simulink model "HvacEvaprTCtrl"  (R2022b)
settings: Solver=FixedStepDiscrete  StartTime=0.0  StopTime=10.0  FixedStep=1  RelTol=1e-3
settings Model: Creator=blue.ge  LastModifiedBy=blue.ge
settings DataIOCC: SaveFormat=StructureWithTime
settings OptimizationCC: DefaultParameterBehavior=Inlined  EnhancedBackFolding=on  PassReuseOutputArgsAs=Individual arguments  ZeroExternalMemoryAtStartup=off  ZeroInternalMemoryAtStartup=off  OptimizeBlockOrder=speed  DifferentSizesBufferReuse=on  OptimizationCustomize=off  EfficientTunableParamExpr=on
settings DebuggingCC: ParameterTunabilityLossMsg=error
settings ModelReferenceCC: ModelReferenceNumInstancesAllowed=Single
settings SFSimCC: MATLABDynamicMemAlloc=off
settings RTWCC: Description=AUTOSAR  SystemTargetFile=autosar.tlc  GenCodeOnly=on  TemplateMakefile=ert_default_tmf
settings CodeAppCC: ForceParamTrailComments=on  IgnoreCustomStorageClasses=off  OperatorAnnotations=on  SimulinkDataObjDesc=on  SFDataObjDesc=on  InsertBlockDesc=on
settings STFCustomTargetCC: TargetLangStandard=C99 (ISO)  MultiwordLength=256  ParMdlRefBuildCompliant=on  CompOptLevelCompliant=on  IncludeMdlTerminateFcn=off  CombineOutputUpdateFcns=on  SuppressErrorStatus=on  ERTCustomFileBanners=on  SupportNonFinite=off  SupportComplex=off  SupportContinuousTime=off  SupportNonInlinedSFcns=off  RemoveResetFunc=on  MATLABClassNameForMDSCustomization=Simulink.SoftwareTarget.ERTCustomization  AutosarCompliant=on  UseToolchainInfoCompliant=on  LookupTableObjectStructAxisOrder=2,1,3,4,...  LUTObjectStructOrderEvenSpacing=Size,Table,Breakpoints  SystemTargetFile=autosar.tlc
settings ERTTargetCC: TargetLangStandard=C99 (ISO)  ConcurrentExecutionCompliant=off  IncludeMdlTerminateFcn=off  SuppressErrorStatus=on  SupportNonFinite=off  SupportComplex=off  ModelStepFunctionPrototypeControlCompliant=off  CPPClassGenCompliant=off  AutosarCompliant=on  LookupTableObjectStructAxisOrder=2,1,3,4,...  LUTObjectStructOrderEvenSpacing=Size,Table,Breakpoints  InlinedParameterPlacement=NonHierarchical  ERTCustomFileTemplate=example_file_process.tlc

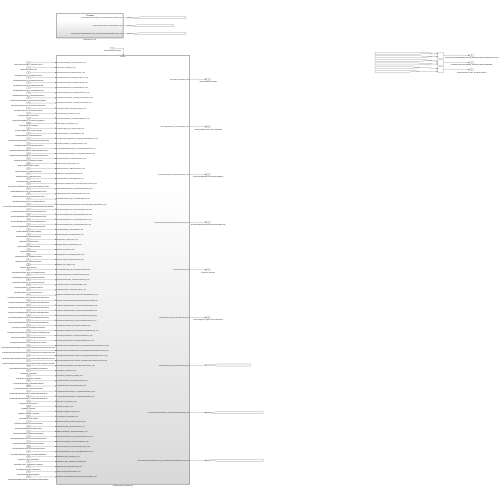
[thumbnail: Simulink's stored preview of the top-level diagram]
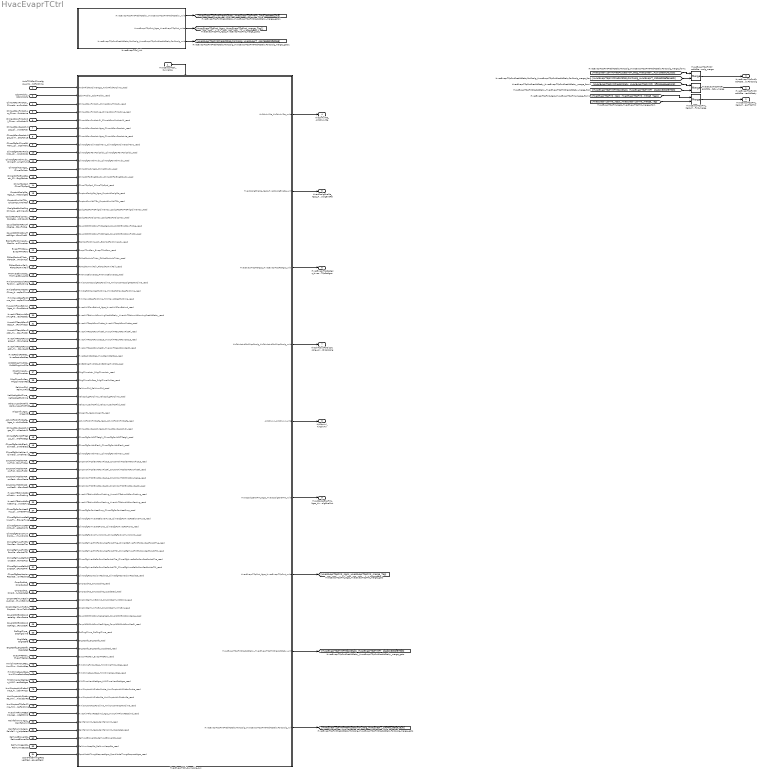
== system HvacEvaprTCtrl ==
  HvacEvaprTCtrl_Runnable : Inport  SampleTime=1  OutputFunctionCall=on
  AmbTFildForClimaNgcc_AmbTFildForClima : Inport  Port=2  OutDataTypeStr=T30  PortDimensions=1
  CdsnProblty_CdsnProblty : Inport  Port=3  OutDataTypeStr=Fac30  PortDimensions=1
  ClimaAtRowFrntActv_ClimaAtRowFrntActv : Inport  Port=4  OutDataTypeStr=Boolean  PortDimensions=1
  ClimaAtRowFrntActvLe_ClimaAtRowFrntActvLe : Inport  Port=5  OutDataTypeStr=Boolean  PortDimensions=1
  ClimaAtRowFrntActvRi_ClimaAtRowFrntActvRi : Inport  Port=6  OutDataTypeStr=Boolean  PortDimensions=1
  ClimaAtRowSecActvNgcc_ClimaAtRowSecActv : Inport  Port=7  OutDataTypeStr=Boolean  PortDimensions=1
  ClimaAtRowSecActvNgcc_ClimaAtRowSecActvLe : Inport  Port=8  OutDataTypeStr=Boolean  PortDimensions=1
  ClimaCfgForClimaCtrlMan1_ClimaCfgForClimaCtrlMan1 : Inport  Port=9  OutDataTypeStr=Boolean  PortDimensions=1
  ClimaCfgForFanPotiCtrld1_ClimaCfgForFanPotiCtrld1 : Inport  Port=10  OutDataTypeStr=Boolean  PortDimensions=1
  ClimaCfgForVehHyb1_ClimaCfgForVehHyb1 : Inport  Port=11  OutDataTypeStr=Boolean  PortDimensions=1
  ClimaCtrlActvNgcc_ClimaCtrlActv : Inport  Port=12  OutDataTypeStr=Boolean  PortDimensions=1
  ClimaCtrlForEngStbActv_ClimaCtrlForEngStbActv : Inport  Port=13  OutDataTypeStr=Boolean  PortDimensions=1
  ClimaTSpSpcl_ClimaTSpSpcl : Inport  Port=14  OutDataTypeStr=Bus: ClimaTSpSpcl  PortDimensions=1  BusOutputAsStruct=on
  CmptmtCoolgSts_Ngcc_CmptmtCoolgSts : Inport  Port=15  OutDataTypeStr=Enum: CmptmtCoolgSts  PortDimensions=1
  CmptmtSunMtrlTErr_CmptmtSunMtrlTErr : Inport  Port=16  OutDataTypeStr=T30  PortDimensions=1
  CoolgFastForPrePrkgClimaActv_CoolgFastForPrePrkgClimaActv : Inport  Port=17  OutDataTypeStr=Boolean  PortDimensions=1
  CoolgReqForDispActv_CoolgReqForDispActv : Inport  Port=18  OutDataTypeStr=Enum: CoolgReqForDisp  PortDimensions=1
  CoupldCtrlErrAtRowFirstLeNgcc_CoupldCtrlErrAtRowFirstLe : Inport  Port=19  OutDataTypeStr=T30  PortDimensions=1
  CoupldCtrlErrAtRowFirstRiNgcc_CoupldCtrlErrAtRowFirstRi : Inport  Port=20  OutDataTypeStr=T30  PortDimensions=1
  EcoModForClimaActv_EcoModForClimaActv : Inport  Port=21  OutDataTypeStr=Enum: OnOff1  PortDimensions=1
  EvaprTFrntRaw_EvaprTFrntRaw : Inport  Port=22  OutDataTypeStr=Bus: EvaprTFrnt  PortDimensions=1  BusOutputAsStruct=on
  FltModForAmbTActv_FltModForAmbTActv : Inport  Port=23  OutDataTypeStr=Boolean  PortDimensions=1
  FltModForHmiFailr_FltModForHmiFailr : Inport  Port=24  OutDataTypeStr=Boolean  PortDimensions=1
  FrntHvacBlowerSts_FrntHvacBlowerSts : Inport  Port=25  OutDataTypeStr=Enum: HvacFanSts  PortDimensions=1
  HmiCmptmtCoolgReqForClima_HmiCmptmtCoolgReqForClima : Inport  Port=26  OutDataTypeStr=Enum: HmiCmptmtCoolgReq  PortDimensions=1
  HmiDefrstMaxReqForClima_HmiDefrstMaxReqForClima : Inport  Port=27  OutDataTypeStr=Enum: ActrReq  PortDimensions=1
  HmiMaxACReqForClima_HmiMaxACReqForClima : Inport  Port=28  OutDataTypeStr=Enum: OnOff1  PortDimensions=1
  HvacAirMFlowEstimd_Ngcc_HvacAirMFlowEstimd : Inport  Port=29  OutMin=0  OutMax=1000  OutDataTypeStr=MFlow1  PortDimensions=1
  HvacAirTEstimnAtRowAvgPredAtStaby_HvacAirTEstimnAtRowAvgPredAtStaby : Inport  Port=30  OutDataTypeStr=T30  PortDimensions=1
  HvacAirTReqAtRowFirstLe_HvacAirTReqAtRowFirstLe : Inport  Port=31  OutDataTypeStr=T30  PortDimensions=1
  HvacAirTReqAtRowFirstRi_HvacAirTReqAtRowFirstRi : Inport  Port=32  OutDataTypeStr=T30  PortDimensions=1
  HvacAirTReqAtRowSecLe_HvacAirTReqAtRowSecLe : Inport  Port=33  OutDataTypeStr=T30  PortDimensions=1
  HvacAirTReqAtRowSecRi_HvacAirTReqAtRowSecRi : Inport  Port=34  OutDataTypeStr=T30  PortDimensions=1
  HvacRecircRatReq_HvacRecircRatReq : Inport  Port=35  OutDataTypeStr=Perc30  PortDimensions=1
  OnBdChrgrHndlSts_OnBdChrgrHndlSts : Inport  Port=36  OutDataTypeStr=Enum: OnBdChrgrSts1  PortDimensions=1
  PrkgClimaActv_PrkgClimaActv : Inport  Port=37  OutDataTypeStr=Enum: PrkgClimaActv  PortDimensions=1
  PrkgClimaOnReq_PrkgClimaOnReq : Inport  Port=38  OutDataTypeStr=Boolean  PortDimensions=1
  RelHumFild_RelHumFild : Inport  Port=39  OutDataTypeStr=Perc7  PortDimensions=1
  VehSpdLgtForClima_VehSpdLgtForClima : Inport  Port=40  OutDataTypeStr=Bus: VehSpdLgtForClima  PortDimensions=1  BusOutputAsStruct=on
  VehSunLoadTotFild_VehSunLoadTotFild : Inport  Port=41  OutDataTypeStr=SunLoad30  PortDimensions=1
  WipgInfo_Ngcc_WipgInfo : Inport  Port=42  OutDataTypeStr=Bus: WipgInfo  PortDimensions=1  BusOutputAsStruct=on
  ActvnOfWshrFrntSafe_Ngcc_ActvnOfWshrFrntSafe : Inport  Port=43  OutDataTypeStr=Enum: OnOffCrit1  PortDimensions=1
  ClimaAtRowSecActvNgcc_ClimaAtRowSecActvRi : Inport  Port=44  OutDataTypeStr=Boolean  PortDimensions=1
  ClimaCfgForNrOfTSetg1_ClimaCfgForNrOfTSetg1 : Inport  Port=45  OutDataTypeStr=Enum: ClimaCfgForNrOfTSetg1  PortDimensions=1
  ClimaCfgForVehElec1_ClimaCfgForVehElec1 : Inport  Port=46  OutDataTypeStr=Boolean  PortDimensions=1
  ClimaCfgForVehHev1_ClimaCfgForVehHev1 : Inport  Port=47  OutDataTypeStr=Boolean  PortDimensions=1
  CmptmtAirTCtrlErrAtRowFirstLe_CmptmtAirTCtrlErrAtRowFirstLe : Inport  Port=48  OutDataTypeStr=T30  PortDimensions=1
  CmptmtAirTCtrlErrAtRowFirstRi_CmptmtAirTCtrlErrAtRowFirstRi : Inport  Port=49  OutDataTypeStr=T30  PortDimensions=1
  CmptmtAirTCtrlErrAtRowSecLe_CmptmtAirTCtrlErrAtRowSecLe : Inport  Port=50  OutDataTypeStr=T30  PortDimensions=1
  CmptmtAirTCtrlErrAtRowSecRi_CmptmtAirTCtrlErrAtRowSecRi : Inport  Port=51  OutDataTypeStr=T30  PortDimensions=1
  HvacAirTEstimdAtRowFirstAvg_HvacAirTEstimdAtRowFirstAvg : Inport  Port=52  OutDataTypeStr=T30  PortDimensions=1
  HvacAirTEstimdAtRowSecAvg_HvacAirTEstimdAtRowSecAvg : Inport  Port=53  OutDataTypeStr=T30  PortDimensions=1
  ClimaCfgForForHeatPmp_ClimaCfgForForHeatPmp : Inport  Port=54  OutDataTypeStr=Enum: CarCfgForHeatPmpTyp  PortDimensions=1
  ClimaCfgForHvacReBlowerType_ClimaCfgForHvacReBlowerType : Inport  Port=55  OutDataTypeStr=Enum: ClimaCfgForHvacReBlowerType  PortDimensions=1
  ClimaCfgForHvacRePrsnt1_ClimaCfgForHvacRePrsnt1 : Inport  Port=56  OutDataTypeStr=Enum: CarCfgForHvacRe  PortDimensions=1
  ClimaCfgForSnsrHumPrsnt1_ClimaCfgForSnsrHumPrsnt1 : Inport  Port=57  OutDataTypeStr=Boolean  PortDimensions=1
  ClimaCfgHvacFrntForRowSecForActrTLe_ClimaCfgHvacFrntForRowSecForActrTLe : Inport  Port=58  OutDataTypeStr=Boolean  PortDimensions=1
  ClimaCfgHvacFrntForRowSecForActrTRi_ClimaCfgHvacFrntForRowSecForActrTRi : Inport  Port=59  OutDataTypeStr=Boolean  PortDimensions=1
  ClimaCfgHvacReForRowSecForActrTLe_ClimaCfgHvacReForRowSecForActrTLe : Inport  Port=60  OutDataTypeStr=UInt8  PortDimensions=1
  ClimaCfgHvacReForRowSecForActrTRi_ClimaCfgHvacReForRowSecForActrTRi : Inport  Port=61  OutDataTypeStr=UInt8  PortDimensions=1
  ClimaCfgReqVersionReplace_ClimaCfgReqVersionReplace : Inport  Port=62  OutDataTypeStr=UInt8  PortDimensions=1
  CmprSpdAct_CmprSpdAct : Inport  Port=63  OutDataTypeStr=CmprSpd1  PortDimensions=1
  CmprSpdAct_CmprSpdAct_IsUpdated : Inport  Port=64  OutDataTypeStr=boolean  PortDimensions=1
  CmptmtRelHumEstimd_CmptmtRelHumEstimd : Inport  Port=65  OutDataTypeStr=Perc7  PortDimensions=1
  CmptmtRelHumToExt_CmptmtRelHumToExt : Inport  Port=66  OutDataTypeStr=Bus: CmptmtRelHumToExt  PortDimensions=1  BusOutputAsStruct=on
  CoupldCtrlErrAtRowSecLeNgcc_CoupldCtrlErrAtRowSecLe : Inport  Port=67  OutDataTypeStr=T30  PortDimensions=1
  CoupldCtrlErrAtRowSecRiNgcc_CoupldCtrlErrAtRowSecRi : Inport  Port=68  OutDataTypeStr=T30  PortDimensions=1
  DsChrgClima_DsChrgClima : Inport  Port=69  OutDataTypeStr=Boolean  PortDimensions=1
  EngNSafe_EngNSafe : Inport  Port=70  OutDataTypeStr=Bus: EngNSafe  PortDimensions=1  BusOutputAsStruct=on
  EngNSafe_EngNSafe_IsUpdated : Inport  Port=71  OutDataTypeStr=boolean  PortDimensions=1
  EvaprTReRaw_EvaprTReRaw : Inport  Port=72  OutDataTypeStr=Bus: EvaprTFrnt  PortDimensions=1  BusOutputAsStruct=on
  HmiClimaFrntAutReq_HmiClimaFrntAutReq : Inport  Port=73  OutDataTypeStr=Enum: OnOff1  PortDimensions=1
  HmiClimaReAutReq_HmiClimaReAutReq : Inport  Port=74  OutDataTypeStr=Enum: OnOff1  PortDimensions=1
  HMIClimaVentSetNgcc_HMIClimaVentSetNgcc : Inport  Port=75  OutDataTypeStr=Enum: OnOff1  PortDimensions=1
  HmiCmptmtAirDistbnFrntLe_HmiCmptmtAirDistbnFrntLe : Inport  Port=76  OutDataTypeStr=Enum: HmiCmptmtAirDistbnFrnt  PortDimensions=1
  HmiCmptmtAirDistbnRe_HmiCmptmtAirDistbnRe : Inport  Port=77  OutDataTypeStr=Enum: HmiCmptmtAirDistbnFrnt  PortDimensions=1
  HmiCmptmtTSpForClima_HmiCmptmtTSpForClima : Inport  Port=78  OutDataTypeStr=Bus: HmiCmptmtTSp  PortDimensions=1  BusOutputAsStruct=on
  HvacAirMFlowReEstimd_Ngcc_HvacAirMFlowReEstimd : Inport  Port=79  OutMin=0  OutMax=1000  OutDataTypeStr=MFlow1  PortDimensions=1
  RainfallAmnt_Ngcc_RainfallAmnt : Inport  Port=80  OutDataTypeStr=Enum: AmntSnsr  PortDimensions=1
  RainfallAmnt_Ngcc_RainfallAmnt_IsUpdated : Inport  Port=81  OutDataTypeStr=boolean  PortDimensions=1
  ReHvacBlowerSts_ReHvacBlowerSts : Inport  Port=82  OutDataTypeStr=Enum: HvacFanSts  PortDimensions=1
  RelHumKeepSts_RelHumKeepSts : Inport  Port=83  OutDataTypeStr=Enum: RelHumKeepSts  PortDimensions=1
  SportModeThmgtRequestNgcc_SportModeThmgtRequestNgcc : Inport  Port=84  OutDataTypeStr=Enum: ThmgtLevel  PortDimensions=1
  HvacEvaprTCtrl_Init : Subsystem
  HvacEvaprTCtrl_Runnable_sys : Subsystem
  HvacEvaprTSpFrntPredAtStabyForCoolg_HvacEvaprTSpFrntPredAtStabyForCoolg_merge : Merge
  HvacEvaprTSpFrntPredAtStaby_HvacEvaprTSpFrntPredAtStaby_merge : Merge
  HvacEvaprTSpFrnt_Ngcc_HvacEvaprTSpFrnt_merge : Merge
  HvacEvaprTSpFrntPredAtStaby_HvacEvaprTSpFrntPredAtStaby : Outport  OutDataTypeStr=HvacEvaprTSp  PortDimensions=1
  HvacEvaprTSpFrnt_Ngcc_HvacEvaprTSpFrnt : Outport  Port=2  OutDataTypeStr=HvacEvaprTSp  PortDimensions=1
  HvacEvaprTSpFrntPredAtStabyForCoolg_HvacEvaprTSpFrntPredAtStabyForCoolg : Outport  Port=3  OutDataTypeStr=HvacEvaprTSp  PortDimensions=1
  AirCdnAvlRe_AirCdnAvlRe : Outport  Port=4  OutDataTypeStr=Boolean  PortDimensions=1
  HvacCoolgEnaRe_Ngcc_HvacCoolgEnaRe : Outport  Port=5  OutDataTypeStr=Enum: OnOff1  PortDimensions=1
  HvacEvaprTSpReNgcc_HvacEvaprTSpReNgcc : Outport  Port=6  OutDataTypeStr=HvacEvaprTSp  PortDimensions=1
  AirCdnActvdForDispCoolg_AirCdnActvdForDispCoolg : Outport  Port=7  OutDataTypeStr=Boolean  PortDimensions=1
  AirCdnAvl_AirCdnAvl : Outport  Port=8  OutDataTypeStr=Boolean  PortDimensions=1
  HvacCoolgEnaFrnt_Ngcc_HvacCoolgEnaFrnt : Outport  Port=9  OutDataTypeStr=Enum: OnOff1  PortDimensions=1
  wires:
  AmbTFildForClimaNgcc_AmbTFildForClima/1 -> HvacEvaprTCtrl_Runnable_sys/1(AmbTFildForClimaNgcc_AmbTFildForClima_read)
  CdsnProblty_CdsnProblty/1 -> HvacEvaprTCtrl_Runnable_sys/2(CdsnProblty_CdsnProblty_read)
  ClimaAtRowFrntActv_ClimaAtRowFrntActv/1 -> HvacEvaprTCtrl_Runnable_sys/3(ClimaAtRowFrntActv_ClimaAtRowFrntActv_read)
  ClimaAtRowFrntActvLe_ClimaAtRowFrntActvLe/1 -> HvacEvaprTCtrl_Runnable_sys/4(ClimaAtRowFrntActvLe_ClimaAtRowFrntActvLe_read)
  ClimaAtRowFrntActvRi_ClimaAtRowFrntActvRi/1 -> HvacEvaprTCtrl_Runnable_sys/5(ClimaAtRowFrntActvRi_ClimaAtRowFrntActvRi_read)
  ClimaAtRowSecActvNgcc_ClimaAtRowSecActv/1 -> HvacEvaprTCtrl_Runnable_sys/6(ClimaAtRowSecActvNgcc_ClimaAtRowSecActv_read)
  ClimaAtRowSecActvNgcc_ClimaAtRowSecActvLe/1 -> HvacEvaprTCtrl_Runnable_sys/7(ClimaAtRowSecActvNgcc_ClimaAtRowSecActvLe_read)
  ClimaCfgForClimaCtrlMan1_ClimaCfgForClimaCtrlMan1/1 -> HvacEvaprTCtrl_Runnable_sys/8(ClimaCfgForClimaCtrlMan1_ClimaCfgForClimaCtrlMan1_read)
  ClimaCfgForFanPotiCtrld1_ClimaCfgForFanPotiCtrld1/1 -> HvacEvaprTCtrl_Runnable_sys/9(ClimaCfgForFanPotiCtrld1_ClimaCfgForFanPotiCtrld1_read)
  ClimaCfgForVehHyb1_ClimaCfgForVehHyb1/1 -> HvacEvaprTCtrl_Runnable_sys/10(ClimaCfgForVehHyb1_ClimaCfgForVehHyb1_read)
  ClimaCtrlActvNgcc_ClimaCtrlActv/1 -> HvacEvaprTCtrl_Runnable_sys/11(ClimaCtrlActvNgcc_ClimaCtrlActv_read)
  ClimaCtrlForEngStbActv_ClimaCtrlForEngStbActv/1 -> HvacEvaprTCtrl_Runnable_sys/12(ClimaCtrlForEngStbActv_ClimaCtrlForEngStbActv_read)
  ClimaTSpSpcl_ClimaTSpSpcl/1 -> HvacEvaprTCtrl_Runnable_sys/13(ClimaTSpSpcl_ClimaTSpSpcl_read)
  CmptmtCoolgSts_Ngcc_CmptmtCoolgSts/1 -> HvacEvaprTCtrl_Runnable_sys/14(CmptmtCoolgSts_Ngcc_CmptmtCoolgSts_read)
  CmptmtSunMtrlTErr_CmptmtSunMtrlTErr/1 -> HvacEvaprTCtrl_Runnable_sys/15(CmptmtSunMtrlTErr_CmptmtSunMtrlTErr_read)
  CoolgFastForPrePrkgClimaActv_CoolgFastForPrePrkgClimaActv/1 -> HvacEvaprTCtrl_Runnable_sys/16(CoolgFastForPrePrkgClimaActv_CoolgFastForPrePrkgClimaActv_read)
  CoolgReqForDispActv_CoolgReqForDispActv/1 -> HvacEvaprTCtrl_Runnable_sys/17(CoolgReqForDispActv_CoolgReqForDispActv_read)
  CoupldCtrlErrAtRowFirstLeNgcc_CoupldCtrlErrAtRowFirstLe/1 -> HvacEvaprTCtrl_Runnable_sys/18(CoupldCtrlErrAtRowFirstLeNgcc_CoupldCtrlErrAtRowFirstLe_read)
  CoupldCtrlErrAtRowFirstRiNgcc_CoupldCtrlErrAtRowFirstRi/1 -> HvacEvaprTCtrl_Runnable_sys/19(CoupldCtrlErrAtRowFirstRiNgcc_CoupldCtrlErrAtRowFirstRi_read)
  EcoModForClimaActv_EcoModForClimaActv/1 -> HvacEvaprTCtrl_Runnable_sys/20(EcoModForClimaActv_EcoModForClimaActv_read)
  EvaprTFrntRaw_EvaprTFrntRaw/1 -> HvacEvaprTCtrl_Runnable_sys/21(EvaprTFrntRaw_EvaprTFrntRaw_read)
  FltModForAmbTActv_FltModForAmbTActv/1 -> HvacEvaprTCtrl_Runnable_sys/22(FltModForAmbTActv_FltModForAmbTActv_read)
  FltModForHmiFailr_FltModForHmiFailr/1 -> HvacEvaprTCtrl_Runnable_sys/23(FltModForHmiFailr_FltModForHmiFailr_read)
  FrntHvacBlowerSts_FrntHvacBlowerSts/1 -> HvacEvaprTCtrl_Runnable_sys/24(FrntHvacBlowerSts_FrntHvacBlowerSts_read)
  HmiCmptmtCoolgReqForClima_HmiCmptmtCoolgReqForClima/1 -> HvacEvaprTCtrl_Runnable_sys/25(HmiCmptmtCoolgReqForClima_HmiCmptmtCoolgReqForClima_read)
  HmiDefrstMaxReqForClima_HmiDefrstMaxReqForClima/1 -> HvacEvaprTCtrl_Runnable_sys/26(HmiDefrstMaxReqForClima_HmiDefrstMaxReqForClima_read)
  HmiMaxACReqForClima_HmiMaxACReqForClima/1 -> HvacEvaprTCtrl_Runnable_sys/27(HmiMaxACReqForClima_HmiMaxACReqForClima_read)
  HvacAirMFlowEstimd_Ngcc_HvacAirMFlowEstimd/1 -> HvacEvaprTCtrl_Runnable_sys/28(HvacAirMFlowEstimd_Ngcc_HvacAirMFlowEstimd_read)
  HvacAirTEstimnAtRowAvgPredAtStaby_HvacAirTEstimnAtRowAvgPredAtStaby/1 -> HvacEvaprTCtrl_Runnable_sys/29(HvacAirTEstimnAtRowAvgPredAtStaby_HvacAirTEstimnAtRowAvgPredAtStaby_read)
  HvacAirTReqAtRowFirstLe_HvacAirTReqAtRowFirstLe/1 -> HvacEvaprTCtrl_Runnable_sys/30(HvacAirTReqAtRowFirstLe_HvacAirTReqAtRowFirstLe_read)
  HvacAirTReqAtRowFirstRi_HvacAirTReqAtRowFirstRi/1 -> HvacEvaprTCtrl_Runnable_sys/31(HvacAirTReqAtRowFirstRi_HvacAirTReqAtRowFirstRi_read)
  HvacAirTReqAtRowSecLe_HvacAirTReqAtRowSecLe/1 -> HvacEvaprTCtrl_Runnable_sys/32(HvacAirTReqAtRowSecLe_HvacAirTReqAtRowSecLe_read)
  HvacAirTReqAtRowSecRi_HvacAirTReqAtRowSecRi/1 -> HvacEvaprTCtrl_Runnable_sys/33(HvacAirTReqAtRowSecRi_HvacAirTReqAtRowSecRi_read)
  HvacRecircRatReq_HvacRecircRatReq/1 -> HvacEvaprTCtrl_Runnable_sys/34(HvacRecircRatReq_HvacRecircRatReq_read)
  OnBdChrgrHndlSts_OnBdChrgrHndlSts/1 -> HvacEvaprTCtrl_Runnable_sys/35(OnBdChrgrHndlSts_OnBdChrgrHndlSts_read)
  PrkgClimaActv_PrkgClimaActv/1 -> HvacEvaprTCtrl_Runnable_sys/36(PrkgClimaActv_PrkgClimaActv_read)
  PrkgClimaOnReq_PrkgClimaOnReq/1 -> HvacEvaprTCtrl_Runnable_sys/37(PrkgClimaOnReq_PrkgClimaOnReq_read)
  RelHumFild_RelHumFild/1 -> HvacEvaprTCtrl_Runnable_sys/38(RelHumFild_RelHumFild_read)
  VehSpdLgtForClima_VehSpdLgtForClima/1 -> HvacEvaprTCtrl_Runnable_sys/39(VehSpdLgtForClima_VehSpdLgtForClima_read)
  VehSunLoadTotFild_VehSunLoadTotFild/1 -> HvacEvaprTCtrl_Runnable_sys/40(VehSunLoadTotFild_VehSunLoadTotFild_read)
  WipgInfo_Ngcc_WipgInfo/1 -> HvacEvaprTCtrl_Runnable_sys/41(WipgInfo_Ngcc_WipgInfo_read)
  ActvnOfWshrFrntSafe_Ngcc_ActvnOfWshrFrntSafe/1 -> HvacEvaprTCtrl_Runnable_sys/42(ActvnOfWshrFrntSafe_Ngcc_ActvnOfWshrFrntSafe_read)
  ClimaAtRowSecActvNgcc_ClimaAtRowSecActvRi/1 -> HvacEvaprTCtrl_Runnable_sys/43(ClimaAtRowSecActvNgcc_ClimaAtRowSecActvRi_read)
  ClimaCfgForNrOfTSetg1_ClimaCfgForNrOfTSetg1/1 -> HvacEvaprTCtrl_Runnable_sys/44(ClimaCfgForNrOfTSetg1_ClimaCfgForNrOfTSetg1_read)
  ClimaCfgForVehElec1_ClimaCfgForVehElec1/1 -> HvacEvaprTCtrl_Runnable_sys/45(ClimaCfgForVehElec1_ClimaCfgForVehElec1_read)
  ClimaCfgForVehHev1_ClimaCfgForVehHev1/1 -> HvacEvaprTCtrl_Runnable_sys/46(ClimaCfgForVehHev1_ClimaCfgForVehHev1_read)
  CmptmtAirTCtrlErrAtRowFirstLe_CmptmtAirTCtrlErrAtRowFirstLe/1 -> HvacEvaprTCtrl_Runnable_sys/47(CmptmtAirTCtrlErrAtRowFirstLe_CmptmtAirTCtrlErrAtRowFirstLe_read)
  CmptmtAirTCtrlErrAtRowFirstRi_CmptmtAirTCtrlErrAtRowFirstRi/1 -> HvacEvaprTCtrl_Runnable_sys/48(CmptmtAirTCtrlErrAtRowFirstRi_CmptmtAirTCtrlErrAtRowFirstRi_read)
  CmptmtAirTCtrlErrAtRowSecLe_CmptmtAirTCtrlErrAtRowSecLe/1 -> HvacEvaprTCtrl_Runnable_sys/49(CmptmtAirTCtrlErrAtRowSecLe_CmptmtAirTCtrlErrAtRowSecLe_read)
  CmptmtAirTCtrlErrAtRowSecRi_CmptmtAirTCtrlErrAtRowSecRi/1 -> HvacEvaprTCtrl_Runnable_sys/50(CmptmtAirTCtrlErrAtRowSecRi_CmptmtAirTCtrlErrAtRowSecRi_read)
  HvacAirTEstimdAtRowFirstAvg_HvacAirTEstimdAtRowFirstAvg/1 -> HvacEvaprTCtrl_Runnable_sys/51(HvacAirTEstimdAtRowFirstAvg_HvacAirTEstimdAtRowFirstAvg_read)
  HvacAirTEstimdAtRowSecAvg_HvacAirTEstimdAtRowSecAvg/1 -> HvacEvaprTCtrl_Runnable_sys/52(HvacAirTEstimdAtRowSecAvg_HvacAirTEstimdAtRowSecAvg_read)
  ClimaCfgForForHeatPmp_ClimaCfgForForHeatPmp/1 -> HvacEvaprTCtrl_Runnable_sys/53(ClimaCfgForForHeatPmp_ClimaCfgForForHeatPmp_read)
  ClimaCfgForHvacReBlowerType_ClimaCfgForHvacReBlowerType/1 -> HvacEvaprTCtrl_Runnable_sys/54(ClimaCfgForHvacReBlowerType_ClimaCfgForHvacReBlowerType_read)
  ClimaCfgForHvacRePrsnt1_ClimaCfgForHvacRePrsnt1/1 -> HvacEvaprTCtrl_Runnable_sys/55(ClimaCfgForHvacRePrsnt1_ClimaCfgForHvacRePrsnt1_read)
  ClimaCfgForSnsrHumPrsnt1_ClimaCfgForSnsrHumPrsnt1/1 -> HvacEvaprTCtrl_Runnable_sys/56(ClimaCfgForSnsrHumPrsnt1_ClimaCfgForSnsrHumPrsnt1_read)
  ClimaCfgHvacFrntForRowSecForActrTLe_ClimaCfgHvacFrntForRowSecForActrTLe/1 -> HvacEvaprTCtrl_Runnable_sys/57(ClimaCfgHvacFrntForRowSecForActrTLe_ClimaCfgHvacFrntForRowSecForActrTLe_read)
  ClimaCfgHvacFrntForRowSecForActrTRi_ClimaCfgHvacFrntForRowSecForActrTRi/1 -> HvacEvaprTCtrl_Runnable_sys/58(ClimaCfgHvacFrntForRowSecForActrTRi_ClimaCfgHvacFrntForRowSecForActrTRi_read)
  ClimaCfgHvacReForRowSecForActrTLe_ClimaCfgHvacReForRowSecForActrTLe/1 -> HvacEvaprTCtrl_Runnable_sys/59(ClimaCfgHvacReForRowSecForActrTLe_ClimaCfgHvacReForRowSecForActrTLe_read)
  ClimaCfgHvacReForRowSecForActrTRi_ClimaCfgHvacReForRowSecForActrTRi/1 -> HvacEvaprTCtrl_Runnable_sys/60(ClimaCfgHvacReForRowSecForActrTRi_ClimaCfgHvacReForRowSecForActrTRi_read)
  ClimaCfgReqVersionReplace_ClimaCfgReqVersionReplace/1 -> HvacEvaprTCtrl_Runnable_sys/61(ClimaCfgReqVersionReplace_ClimaCfgReqVersionReplace_read)
  CmprSpdAct_CmprSpdAct/1 -> HvacEvaprTCtrl_Runnable_sys/62(CmprSpdAct_CmprSpdAct_read)
  CmprSpdAct_CmprSpdAct_IsUpdated/1 -> HvacEvaprTCtrl_Runnable_sys/63(CmprSpdAct_CmprSpdAct_IsUpdated_read)
  CmptmtRelHumEstimd_CmptmtRelHumEstimd/1 -> HvacEvaprTCtrl_Runnable_sys/64(CmptmtRelHumEstimd_CmptmtRelHumEstimd_read)
  CmptmtRelHumToExt_CmptmtRelHumToExt/1 -> HvacEvaprTCtrl_Runnable_sys/65(CmptmtRelHumToExt_CmptmtRelHumToExt_read)
  CoupldCtrlErrAtRowSecLeNgcc_CoupldCtrlErrAtRowSecLe/1 -> HvacEvaprTCtrl_Runnable_sys/66(CoupldCtrlErrAtRowSecLeNgcc_CoupldCtrlErrAtRowSecLe_read)
  CoupldCtrlErrAtRowSecRiNgcc_CoupldCtrlErrAtRowSecRi/1 -> HvacEvaprTCtrl_Runnable_sys/67(CoupldCtrlErrAtRowSecRiNgcc_CoupldCtrlErrAtRowSecRi_read)
  DsChrgClima_DsChrgClima/1 -> HvacEvaprTCtrl_Runnable_sys/68(DsChrgClima_DsChrgClima_read)
  EngNSafe_EngNSafe/1 -> HvacEvaprTCtrl_Runnable_sys/69(EngNSafe_EngNSafe_read)
  EngNSafe_EngNSafe_IsUpdated/1 -> HvacEvaprTCtrl_Runnable_sys/70(EngNSafe_EngNSafe_IsUpdated_read)
  EvaprTReRaw_EvaprTReRaw/1 -> HvacEvaprTCtrl_Runnable_sys/71(EvaprTReRaw_EvaprTReRaw_read)
  HmiClimaFrntAutReq_HmiClimaFrntAutReq/1 -> HvacEvaprTCtrl_Runnable_sys/72(HmiClimaFrntAutReq_HmiClimaFrntAutReq_read)
  HmiClimaReAutReq_HmiClimaReAutReq/1 -> HvacEvaprTCtrl_Runnable_sys/73(HmiClimaReAutReq_HmiClimaReAutReq_read)
  HMIClimaVentSetNgcc_HMIClimaVentSetNgcc/1 -> HvacEvaprTCtrl_Runnable_sys/74(HMIClimaVentSetNgcc_HMIClimaVentSetNgcc_read)
  HmiCmptmtAirDistbnFrntLe_HmiCmptmtAirDistbnFrntLe/1 -> HvacEvaprTCtrl_Runnable_sys/75(HmiCmptmtAirDistbnFrntLe_HmiCmptmtAirDistbnFrntLe_read)
  HmiCmptmtAirDistbnRe_HmiCmptmtAirDistbnRe/1 -> HvacEvaprTCtrl_Runnable_sys/76(HmiCmptmtAirDistbnRe_HmiCmptmtAirDistbnRe_read)
  HmiCmptmtTSpForClima_HmiCmptmtTSpForClima/1 -> HvacEvaprTCtrl_Runnable_sys/77(HmiCmptmtTSpForClima_HmiCmptmtTSpForClima_read)
  HvacAirMFlowReEstimd_Ngcc_HvacAirMFlowReEstimd/1 -> HvacEvaprTCtrl_Runnable_sys/78(HvacAirMFlowReEstimd_Ngcc_HvacAirMFlowReEstimd_read)
  RainfallAmnt_Ngcc_RainfallAmnt/1 -> HvacEvaprTCtrl_Runnable_sys/79(RainfallAmnt_Ngcc_RainfallAmnt_read)
  RainfallAmnt_Ngcc_RainfallAmnt_IsUpdated/1 -> HvacEvaprTCtrl_Runnable_sys/80(RainfallAmnt_Ngcc_RainfallAmnt_IsUpdated_read)
  ReHvacBlowerSts_ReHvacBlowerSts/1 -> HvacEvaprTCtrl_Runnable_sys/81(ReHvacBlowerSts_ReHvacBlowerSts_read)
  RelHumKeepSts_RelHumKeepSts/1 -> HvacEvaprTCtrl_Runnable_sys/82(RelHumKeepSts_RelHumKeepSts_read)
  SportModeThmgtRequestNgcc_SportModeThmgtRequestNgcc/1 -> HvacEvaprTCtrl_Runnable_sys/83(SportModeThmgtRequestNgcc_SportModeThmgtRequestNgcc_read)
  HvacEvaprTCtrl_Runnable_sys/1(AirCdnAvlRe_AirCdnAvlRe_write) -> AirCdnAvlRe_AirCdnAvlRe/1
  HvacEvaprTCtrl_Runnable_sys/2(HvacCoolgEnaRe_Ngcc_HvacCoolgEnaRe_write) -> HvacCoolgEnaRe_Ngcc_HvacCoolgEnaRe/1
  HvacEvaprTCtrl_Runnable_sys/3(HvacEvaprTSpReNgcc_HvacEvaprTSpReNgcc_write) -> HvacEvaprTSpReNgcc_HvacEvaprTSpReNgcc/1
  HvacEvaprTCtrl_Runnable_sys/4(AirCdnActvdForDispCoolg_AirCdnActvdForDispCoolg_write) -> AirCdnActvdForDispCoolg_AirCdnActvdForDispCoolg/1
  HvacEvaprTCtrl_Runnable_sys/5(AirCdnAvl_AirCdnAvl_write) -> AirCdnAvl_AirCdnAvl/1
  HvacEvaprTCtrl_Runnable_sys/6(HvacCoolgEnaFrnt_Ngcc_HvacCoolgEnaFrnt_write) -> HvacCoolgEnaFrnt_Ngcc_HvacCoolgEnaFrnt/1
  HvacEvaprTSpFrnt_Ngcc_HvacEvaprTSpFrnt_merge/1 -> HvacEvaprTSpFrnt_Ngcc_HvacEvaprTSpFrnt/1
  HvacEvaprTSpFrntPredAtStaby_HvacEvaprTSpFrntPredAtStaby_merge/1 -> HvacEvaprTSpFrntPredAtStaby_HvacEvaprTSpFrntPredAtStaby/1
  HvacEvaprTSpFrntPredAtStabyForCoolg_HvacEvaprTSpFrntPredAtStabyForCoolg_merge/1 -> HvacEvaprTSpFrntPredAtStabyForCoolg_HvacEvaprTSpFrntPredAtStabyForCoolg/1
  HvacEvaprTCtrl_Runnable_sys/9(HvacEvaprTSpFrntPredAtStabyForCoolg_HvacEvaprTSpFrntPredAtStabyForCoolg_write) -> [HvacEvaprTSpFrntPredAtStabyForCoolg_HvacEvaprT_c9046028ef5e1e3b]
  [HvacEvaprTSpFrntPredAtStabyForCoolg_HvacEvaprT_c9046028ef5e1e3b] -> HvacEvaprTSpFrntPredAtStabyForCoolg_HvacEvaprTSpFrntPredAtStabyForCoolg_merge/2
  HvacEvaprTCtrl_Init/3(HvacEvaprTSpFrntPredAtStabyForCoolg_HvacEvaprTSpFrntPredAtStabyForCoolg_write) -> [HvacEvaprTSpFrntPredAtStabyForCoolg_HvacEvaprT_41a75a8b91f62b58]
  [HvacEvaprTSpFrntPredAtStabyForCoolg_HvacEvaprT_41a75a8b91f62b58] -> HvacEvaprTSpFrntPredAtStabyForCoolg_HvacEvaprTSpFrntPredAtStabyForCoolg_merge/1
  HvacEvaprTCtrl_Runnable_sys/8(HvacEvaprTSpFrntPredAtStaby_HvacEvaprTSpFrntPredAtStaby_write) -> [HvacEvaprTSpFrntPredAtStaby_HvacEvaprTSpFrntPr_1bad2c6002f879d3]
  [HvacEvaprTSpFrntPredAtStaby_HvacEvaprTSpFrntPr_1bad2c6002f879d3] -> HvacEvaprTSpFrntPredAtStaby_HvacEvaprTSpFrntPredAtStaby_merge/2
  HvacEvaprTCtrl_Init/1(HvacEvaprTSpFrntPredAtStaby_HvacEvaprTSpFrntPredAtStaby_write) -> [HvacEvaprTSpFrntPredAtStaby_HvacEvaprTSpFrntPr_1ffc61aabaee10c8]
  [HvacEvaprTSpFrntPredAtStaby_HvacEvaprTSpFrntPr_1ffc61aabaee10c8] -> HvacEvaprTSpFrntPredAtStaby_HvacEvaprTSpFrntPredAtStaby_merge/1
  HvacEvaprTCtrl_Runnable_sys/7(HvacEvaprTSpFrnt_Ngcc_HvacEvaprTSpFrnt_write) -> [HvacEvaprTSpFrnt_Ngcc_HvacEvaprTSpFrnt_merge_Tag]
  [HvacEvaprTSpFrnt_Ngcc_HvacEvaprTSpFrnt_merge_Tag] -> HvacEvaprTSpFrnt_Ngcc_HvacEvaprTSpFrnt_merge/2
  HvacEvaprTCtrl_Init/2(HvacEvaprTSpFrnt_Ngcc_HvacEvaprTSpFrnt_write) -> [HvacEvaprTSpFrnt_Ngcc_HvacEvaprTSpFrnt_merge_Tag1]
  [HvacEvaprTSpFrnt_Ngcc_HvacEvaprTSpFrnt_merge_Tag1] -> HvacEvaprTSpFrnt_Ngcc_HvacEvaprTSpFrnt_merge/1
  HvacEvaprTCtrl_Runnable/1 -> HvacEvaprTCtrl_Runnable_sys/Trigger

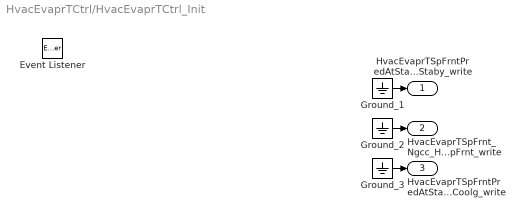
== system HvacEvaprTCtrl/HvacEvaprTCtrl_Init ==
  Event Listener : EventListener  EventName=reset
  Ground_1 : Ground
  Ground_2 : Ground
  Ground_3 : Ground
  HvacEvaprTSpFrntPredAtStaby_HvacEvaprTSpFrntPredAtStaby_write : Outport
  HvacEvaprTSpFrnt_Ngcc_HvacEvaprTSpFrnt_write : Outport  Port=2
  HvacEvaprTSpFrntPredAtStabyForCoolg_HvacEvaprTSpFrntPredAtStabyForCoolg_write : Outport  Port=3
  wires:
  Ground_1/1 -> HvacEvaprTSpFrntPredAtStaby_HvacEvaprTSpFrntPredAtStaby_write/1
  Ground_2/1 -> HvacEvaprTSpFrnt_Ngcc_HvacEvaprTSpFrnt_write/1
  Ground_3/1 -> HvacEvaprTSpFrntPredAtStabyForCoolg_HvacEvaprTSpFrntPredAtStabyForCoolg_write/1

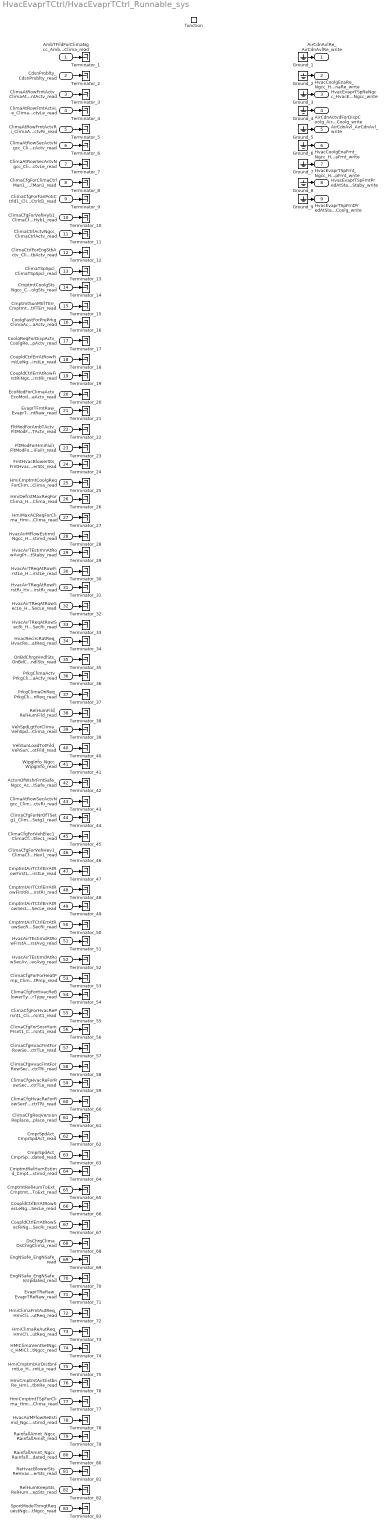
== system HvacEvaprTCtrl/HvacEvaprTCtrl_Runnable_sys ==
  AmbTFildForClimaNgcc_AmbTFildForClima_read : Inport
  CdsnProblty_CdsnProblty_read : Inport  Port=2
  ClimaAtRowFrntActv_ClimaAtRowFrntActv_read : Inport  Port=3
  ClimaAtRowFrntActvLe_ClimaAtRowFrntActvLe_read : Inport  Port=4
  ClimaAtRowFrntActvRi_ClimaAtRowFrntActvRi_read : Inport  Port=5
  ClimaAtRowSecActvNgcc_ClimaAtRowSecActv_read : Inport  Port=6
  ClimaAtRowSecActvNgcc_ClimaAtRowSecActvLe_read : Inport  Port=7
  ClimaCfgForClimaCtrlMan1_ClimaCfgForClimaCtrlMan1_read : Inport  Port=8
  ClimaCfgForFanPotiCtrld1_ClimaCfgForFanPotiCtrld1_read : Inport  Port=9
  ClimaCfgForVehHyb1_ClimaCfgForVehHyb1_read : Inport  Port=10
  ClimaCtrlActvNgcc_ClimaCtrlActv_read : Inport  Port=11
  ClimaCtrlForEngStbActv_ClimaCtrlForEngStbActv_read : Inport  Port=12
  ClimaTSpSpcl_ClimaTSpSpcl_read : Inport  Port=13
  CmptmtCoolgSts_Ngcc_CmptmtCoolgSts_read : Inport  Port=14
  CmptmtSunMtrlTErr_CmptmtSunMtrlTErr_read : Inport  Port=15
  CoolgFastForPrePrkgClimaActv_CoolgFastForPrePrkgClimaActv_read : Inport  Port=16
  CoolgReqForDispActv_CoolgReqForDispActv_read : Inport  Port=17
  CoupldCtrlErrAtRowFirstLeNgcc_CoupldCtrlErrAtRowFirstLe_read : Inport  Port=18
  CoupldCtrlErrAtRowFirstRiNgcc_CoupldCtrlErrAtRowFirstRi_read : Inport  Port=19
  EcoModForClimaActv_EcoModForClimaActv_read : Inport  Port=20
  EvaprTFrntRaw_EvaprTFrntRaw_read : Inport  Port=21
  FltModForAmbTActv_FltModForAmbTActv_read : Inport  Port=22
  FltModForHmiFailr_FltModForHmiFailr_read : Inport  Port=23
  FrntHvacBlowerSts_FrntHvacBlowerSts_read : Inport  Port=24
  HmiCmptmtCoolgReqForClima_HmiCmptmtCoolgReqForClima_read : Inport  Port=25
  HmiDefrstMaxReqForClima_HmiDefrstMaxReqForClima_read : Inport  Port=26
  HmiMaxACReqForClima_HmiMaxACReqForClima_read : Inport  Port=27
  HvacAirMFlowEstimd_Ngcc_HvacAirMFlowEstimd_read : Inport  Port=28
  HvacAirTEstimnAtRowAvgPredAtStaby_HvacAirTEstimnAtRowAvgPredAtStaby_read : Inport  Port=29
  HvacAirTReqAtRowFirstLe_HvacAirTReqAtRowFirstLe_read : Inport  Port=30
  HvacAirTReqAtRowFirstRi_HvacAirTReqAtRowFirstRi_read : Inport  Port=31
  HvacAirTReqAtRowSecLe_HvacAirTReqAtRowSecLe_read : Inport  Port=32
  HvacAirTReqAtRowSecRi_HvacAirTReqAtRowSecRi_read : Inport  Port=33
  HvacRecircRatReq_HvacRecircRatReq_read : Inport  Port=34
  OnBdChrgrHndlSts_OnBdChrgrHndlSts_read : Inport  Port=35
  PrkgClimaActv_PrkgClimaActv_read : Inport  Port=36
  PrkgClimaOnReq_PrkgClimaOnReq_read : Inport  Port=37
  RelHumFild_RelHumFild_read : Inport  Port=38
  VehSpdLgtForClima_VehSpdLgtForClima_read : Inport  Port=39
  VehSunLoadTotFild_VehSunLoadTotFild_read : Inport  Port=40
  WipgInfo_Ngcc_WipgInfo_read : Inport  Port=41
  ActvnOfWshrFrntSafe_Ngcc_ActvnOfWshrFrntSafe_read : Inport  Port=42
  ClimaAtRowSecActvNgcc_ClimaAtRowSecActvRi_read : Inport  Port=43
  ClimaCfgForNrOfTSetg1_ClimaCfgForNrOfTSetg1_read : Inport  Port=44
  ClimaCfgForVehElec1_ClimaCfgForVehElec1_read : Inport  Port=45
  ClimaCfgForVehHev1_ClimaCfgForVehHev1_read : Inport  Port=46
  CmptmtAirTCtrlErrAtRowFirstLe_CmptmtAirTCtrlErrAtRowFirstLe_read : Inport  Port=47
  CmptmtAirTCtrlErrAtRowFirstRi_CmptmtAirTCtrlErrAtRowFirstRi_read : Inport  Port=48
  CmptmtAirTCtrlErrAtRowSecLe_CmptmtAirTCtrlErrAtRowSecLe_read : Inport  Port=49
  CmptmtAirTCtrlErrAtRowSecRi_CmptmtAirTCtrlErrAtRowSecRi_read : Inport  Port=50
  HvacAirTEstimdAtRowFirstAvg_HvacAirTEstimdAtRowFirstAvg_read : Inport  Port=51
  HvacAirTEstimdAtRowSecAvg_HvacAirTEstimdAtRowSecAvg_read : Inport  Port=52
  ClimaCfgForForHeatPmp_ClimaCfgForForHeatPmp_read : Inport  Port=53
  ClimaCfgForHvacReBlowerType_ClimaCfgForHvacReBlowerType_read : Inport  Port=54
  ClimaCfgForHvacRePrsnt1_ClimaCfgForHvacRePrsnt1_read : Inport  Port=55
  ClimaCfgForSnsrHumPrsnt1_ClimaCfgForSnsrHumPrsnt1_read : Inport  Port=56
  ClimaCfgHvacFrntForRowSecForActrTLe_ClimaCfgHvacFrntForRowSecForActrTLe_read : Inport  Port=57
  ClimaCfgHvacFrntForRowSecForActrTRi_ClimaCfgHvacFrntForRowSecForActrTRi_read : Inport  Port=58
  ClimaCfgHvacReForRowSecForActrTLe_ClimaCfgHvacReForRowSecForActrTLe_read : Inport  Port=59
  ClimaCfgHvacReForRowSecForActrTRi_ClimaCfgHvacReForRowSecForActrTRi_read : Inport  Port=60
  ClimaCfgReqVersionReplace_ClimaCfgReqVersionReplace_read : Inport  Port=61
  CmprSpdAct_CmprSpdAct_read : Inport  Port=62
  CmprSpdAct_CmprSpdAct_IsUpdated_read : Inport  Port=63
  CmptmtRelHumEstimd_CmptmtRelHumEstimd_read : Inport  Port=64
  CmptmtRelHumToExt_CmptmtRelHumToExt_read : Inport  Port=65
  CoupldCtrlErrAtRowSecLeNgcc_CoupldCtrlErrAtRowSecLe_read : Inport  Port=66
  CoupldCtrlErrAtRowSecRiNgcc_CoupldCtrlErrAtRowSecRi_read : Inport  Port=67
  DsChrgClima_DsChrgClima_read : Inport  Port=68
  EngNSafe_EngNSafe_read : Inport  Port=69
  EngNSafe_EngNSafe_IsUpdated_read : Inport  Port=70
  EvaprTReRaw_EvaprTReRaw_read : Inport  Port=71
  HmiClimaFrntAutReq_HmiClimaFrntAutReq_read : Inport  Port=72
  HmiClimaReAutReq_HmiClimaReAutReq_read : Inport  Port=73
  HMIClimaVentSetNgcc_HMIClimaVentSetNgcc_read : Inport  Port=74
  HmiCmptmtAirDistbnFrntLe_HmiCmptmtAirDistbnFrntLe_read : Inport  Port=75
  HmiCmptmtAirDistbnRe_HmiCmptmtAirDistbnRe_read : Inport  Port=76
  HmiCmptmtTSpForClima_HmiCmptmtTSpForClima_read : Inport  Port=77
  HvacAirMFlowReEstimd_Ngcc_HvacAirMFlowReEstimd_read : Inport  Port=78
  RainfallAmnt_Ngcc_RainfallAmnt_read : Inport  Port=79
  RainfallAmnt_Ngcc_RainfallAmnt_IsUpdated_read : Inport  Port=80
  ReHvacBlowerSts_ReHvacBlowerSts_read : Inport  Port=81
  RelHumKeepSts_RelHumKeepSts_read : Inport  Port=82
  SportModeThmgtRequestNgcc_SportModeThmgtRequestNgcc_read : Inport  Port=83
  function : TriggerPort  TriggerType=function-call  VariantControl=(inherit)  SampleTimeType=periodic  SampleTime=-1
  Ground_{1..9} (9 alike) : Ground
  Terminator_{1..83} (83 alike) : Terminator
  AirCdnAvlRe_AirCdnAvlRe_write : Outport
      settings: EnsureOutportIsVirtual=on
  HvacCoolgEnaRe_Ngcc_HvacCoolgEnaRe_write : Outport  Port=2
      settings: EnsureOutportIsVirtual=on
  HvacEvaprTSpReNgcc_HvacEvaprTSpReNgcc_write : Outport  Port=3
      settings: EnsureOutportIsVirtual=on
  AirCdnActvdForDispCoolg_AirCdnActvdForDispCoolg_write : Outport  Port=4
      settings: EnsureOutportIsVirtual=on
  AirCdnAvl_AirCdnAvl_write : Outport  Port=5
      settings: EnsureOutportIsVirtual=on
  HvacCoolgEnaFrnt_Ngcc_HvacCoolgEnaFrnt_write : Outport  Port=6
      settings: EnsureOutportIsVirtual=on
  HvacEvaprTSpFrnt_Ngcc_HvacEvaprTSpFrnt_write : Outport  Port=7
      settings: EnsureOutportIsVirtual=on
  HvacEvaprTSpFrntPredAtStaby_HvacEvaprTSpFrntPredAtStaby_write : Outport  Port=8
      settings: EnsureOutportIsVirtual=on
  HvacEvaprTSpFrntPredAtStabyForCoolg_HvacEvaprTSpFrntPredAtStabyForCoolg_write : Outport  Port=9
      settings: EnsureOutportIsVirtual=on
  wires:
  AmbTFildForClimaNgcc_AmbTFildForClima_read/1 -> Terminator_1/1
  CdsnProblty_CdsnProblty_read/1 -> Terminator_2/1
  ClimaAtRowFrntActv_ClimaAtRowFrntActv_read/1 -> Terminator_3/1
  ClimaAtRowFrntActvLe_ClimaAtRowFrntActvLe_read/1 -> Terminator_4/1
  ClimaAtRowFrntActvRi_ClimaAtRowFrntActvRi_read/1 -> Terminator_5/1
  ClimaAtRowSecActvNgcc_ClimaAtRowSecActv_read/1 -> Terminator_6/1
  ClimaAtRowSecActvNgcc_ClimaAtRowSecActvLe_read/1 -> Terminator_7/1
  ClimaCfgForClimaCtrlMan1_ClimaCfgForClimaCtrlMan1_read/1 -> Terminator_8/1
  ClimaCfgForFanPotiCtrld1_ClimaCfgForFanPotiCtrld1_read/1 -> Terminator_9/1
  ClimaCfgForVehHyb1_ClimaCfgForVehHyb1_read/1 -> Terminator_10/1
  ClimaCtrlActvNgcc_ClimaCtrlActv_read/1 -> Terminator_11/1
  ClimaCtrlForEngStbActv_ClimaCtrlForEngStbActv_read/1 -> Terminator_12/1
  ClimaTSpSpcl_ClimaTSpSpcl_read/1 -> Terminator_13/1
  CmptmtCoolgSts_Ngcc_CmptmtCoolgSts_read/1 -> Terminator_14/1
  CmptmtSunMtrlTErr_CmptmtSunMtrlTErr_read/1 -> Terminator_15/1
  CoolgFastForPrePrkgClimaActv_CoolgFastForPrePrkgClimaActv_read/1 -> Terminator_16/1
  CoolgReqForDispActv_CoolgReqForDispActv_read/1 -> Terminator_17/1
  CoupldCtrlErrAtRowFirstLeNgcc_CoupldCtrlErrAtRowFirstLe_read/1 -> Terminator_18/1
  CoupldCtrlErrAtRowFirstRiNgcc_CoupldCtrlErrAtRowFirstRi_read/1 -> Terminator_19/1
  EcoModForClimaActv_EcoModForClimaActv_read/1 -> Terminator_20/1
  EvaprTFrntRaw_EvaprTFrntRaw_read/1 -> Terminator_21/1
  FltModForAmbTActv_FltModForAmbTActv_read/1 -> Terminator_22/1
  FltModForHmiFailr_FltModForHmiFailr_read/1 -> Terminator_23/1
  FrntHvacBlowerSts_FrntHvacBlowerSts_read/1 -> Terminator_24/1
  HmiCmptmtCoolgReqForClima_HmiCmptmtCoolgReqForClima_read/1 -> Terminator_25/1
  HmiDefrstMaxReqForClima_HmiDefrstMaxReqForClima_read/1 -> Terminator_26/1
  HmiMaxACReqForClima_HmiMaxACReqForClima_read/1 -> Terminator_27/1
  HvacAirMFlowEstimd_Ngcc_HvacAirMFlowEstimd_read/1 -> Terminator_28/1
  HvacAirTEstimnAtRowAvgPredAtStaby_HvacAirTEstimnAtRowAvgPredAtStaby_read/1 -> Terminator_29/1
  HvacAirTReqAtRowFirstLe_HvacAirTReqAtRowFirstLe_read/1 -> Terminator_30/1
  HvacAirTReqAtRowFirstRi_HvacAirTReqAtRowFirstRi_read/1 -> Terminator_31/1
  HvacAirTReqAtRowSecLe_HvacAirTReqAtRowSecLe_read/1 -> Terminator_32/1
  HvacAirTReqAtRowSecRi_HvacAirTReqAtRowSecRi_read/1 -> Terminator_33/1
  HvacRecircRatReq_HvacRecircRatReq_read/1 -> Terminator_34/1
  OnBdChrgrHndlSts_OnBdChrgrHndlSts_read/1 -> Terminator_35/1
  PrkgClimaActv_PrkgClimaActv_read/1 -> Terminator_36/1
  PrkgClimaOnReq_PrkgClimaOnReq_read/1 -> Terminator_37/1
  RelHumFild_RelHumFild_read/1 -> Terminator_38/1
  VehSpdLgtForClima_VehSpdLgtForClima_read/1 -> Terminator_39/1
  VehSunLoadTotFild_VehSunLoadTotFild_read/1 -> Terminator_40/1
  WipgInfo_Ngcc_WipgInfo_read/1 -> Terminator_41/1
  ActvnOfWshrFrntSafe_Ngcc_ActvnOfWshrFrntSafe_read/1 -> Terminator_42/1
  ClimaAtRowSecActvNgcc_ClimaAtRowSecActvRi_read/1 -> Terminator_43/1
  ClimaCfgForNrOfTSetg1_ClimaCfgForNrOfTSetg1_read/1 -> Terminator_44/1
  ClimaCfgForVehElec1_ClimaCfgForVehElec1_read/1 -> Terminator_45/1
  ClimaCfgForVehHev1_ClimaCfgForVehHev1_read/1 -> Terminator_46/1
  CmptmtAirTCtrlErrAtRowFirstLe_CmptmtAirTCtrlErrAtRowFirstLe_read/1 -> Terminator_47/1
  CmptmtAirTCtrlErrAtRowFirstRi_CmptmtAirTCtrlErrAtRowFirstRi_read/1 -> Terminator_48/1
  CmptmtAirTCtrlErrAtRowSecLe_CmptmtAirTCtrlErrAtRowSecLe_read/1 -> Terminator_49/1
  CmptmtAirTCtrlErrAtRowSecRi_CmptmtAirTCtrlErrAtRowSecRi_read/1 -> Terminator_50/1
  HvacAirTEstimdAtRowFirstAvg_HvacAirTEstimdAtRowFirstAvg_read/1 -> Terminator_51/1
  HvacAirTEstimdAtRowSecAvg_HvacAirTEstimdAtRowSecAvg_read/1 -> Terminator_52/1
  ClimaCfgForForHeatPmp_ClimaCfgForForHeatPmp_read/1 -> Terminator_53/1
  ClimaCfgForHvacReBlowerType_ClimaCfgForHvacReBlowerType_read/1 -> Terminator_54/1
  ClimaCfgForHvacRePrsnt1_ClimaCfgForHvacRePrsnt1_read/1 -> Terminator_55/1
  ClimaCfgForSnsrHumPrsnt1_ClimaCfgForSnsrHumPrsnt1_read/1 -> Terminator_56/1
  ClimaCfgHvacFrntForRowSecForActrTLe_ClimaCfgHvacFrntForRowSecForActrTLe_read/1 -> Terminator_57/1
  ClimaCfgHvacFrntForRowSecForActrTRi_ClimaCfgHvacFrntForRowSecForActrTRi_read/1 -> Terminator_58/1
  ClimaCfgHvacReForRowSecForActrTLe_ClimaCfgHvacReForRowSecForActrTLe_read/1 -> Terminator_59/1
  ClimaCfgHvacReForRowSecForActrTRi_ClimaCfgHvacReForRowSecForActrTRi_read/1 -> Terminator_60/1
  ClimaCfgReqVersionReplace_ClimaCfgReqVersionReplace_read/1 -> Terminator_61/1
  CmprSpdAct_CmprSpdAct_read/1 -> Terminator_62/1
  CmprSpdAct_CmprSpdAct_IsUpdated_read/1 -> Terminator_63/1
  CmptmtRelHumEstimd_CmptmtRelHumEstimd_read/1 -> Terminator_64/1
  CmptmtRelHumToExt_CmptmtRelHumToExt_read/1 -> Terminator_65/1
  CoupldCtrlErrAtRowSecLeNgcc_CoupldCtrlErrAtRowSecLe_read/1 -> Terminator_66/1
  CoupldCtrlErrAtRowSecRiNgcc_CoupldCtrlErrAtRowSecRi_read/1 -> Terminator_67/1
  DsChrgClima_DsChrgClima_read/1 -> Terminator_68/1
  EngNSafe_EngNSafe_read/1 -> Terminator_69/1
  EngNSafe_EngNSafe_IsUpdated_read/1 -> Terminator_70/1
  EvaprTReRaw_EvaprTReRaw_read/1 -> Terminator_71/1
  HmiClimaFrntAutReq_HmiClimaFrntAutReq_read/1 -> Terminator_72/1
  HmiClimaReAutReq_HmiClimaReAutReq_read/1 -> Terminator_73/1
  HMIClimaVentSetNgcc_HMIClimaVentSetNgcc_read/1 -> Terminator_74/1
  HmiCmptmtAirDistbnFrntLe_HmiCmptmtAirDistbnFrntLe_read/1 -> Terminator_75/1
  HmiCmptmtAirDistbnRe_HmiCmptmtAirDistbnRe_read/1 -> Terminator_76/1
  HmiCmptmtTSpForClima_HmiCmptmtTSpForClima_read/1 -> Terminator_77/1
  HvacAirMFlowReEstimd_Ngcc_HvacAirMFlowReEstimd_read/1 -> Terminator_78/1
  RainfallAmnt_Ngcc_RainfallAmnt_read/1 -> Terminator_79/1
  RainfallAmnt_Ngcc_RainfallAmnt_IsUpdated_read/1 -> Terminator_80/1
  ReHvacBlowerSts_ReHvacBlowerSts_read/1 -> Terminator_81/1
  RelHumKeepSts_RelHumKeepSts_read/1 -> Terminator_82/1
  SportModeThmgtRequestNgcc_SportModeThmgtRequestNgcc_read/1 -> Terminator_83/1
  Ground_1/1 -> AirCdnAvlRe_AirCdnAvlRe_write/1
  Ground_2/1 -> HvacCoolgEnaRe_Ngcc_HvacCoolgEnaRe_write/1
  Ground_3/1 -> HvacEvaprTSpReNgcc_HvacEvaprTSpReNgcc_write/1
  Ground_4/1 -> AirCdnActvdForDispCoolg_AirCdnActvdForDispCoolg_write/1
  Ground_5/1 -> AirCdnAvl_AirCdnAvl_write/1
  Ground_6/1 -> HvacCoolgEnaFrnt_Ngcc_HvacCoolgEnaFrnt_write/1
  Ground_7/1 -> HvacEvaprTSpFrnt_Ngcc_HvacEvaprTSpFrnt_write/1
  Ground_8/1 -> HvacEvaprTSpFrntPredAtStaby_HvacEvaprTSpFrntPredAtStaby_write/1
  Ground_9/1 -> HvacEvaprTSpFrntPredAtStabyForCoolg_HvacEvaprTSpFrntPredAtStabyForCoolg_write/1
